simulink model "BaseEngineController_A02_bak2"  (Simulink 7.7)
settings: Solver=FixedStepDiscrete  StartTime=0.0  StopTime=10.0  RelTol=1e-3
PreLoadFcn:
  | try, eval([bdroot '_setup']); catch, eval('model_setup', ''); end
InitFcn:
  | try, eval([bdroot '_setup']); catch, eval('model_setup', ''); end
undefined symbols (not defined in the file or in its folder): ECU555, High, motohawk_build_version, motohawk_trunc_name, motohawk_vardec_config_group, motohawk_vardec_path, True
libraries: MotoHawk_lib x1081, AutoDoc_lib x609, motohawk_control_primitives_lib x132, motohawk_engine_lib x26, motohawk_annotations_lib x9, simulink x8, motohawk_control_primitives_motoshare_lib x1, simulink_extras x1
(part 6 of 22)

== system BaseEngineController_A02_bak2/Foreground/Control/VirtualSensors/AirCalcs ==
  RawIndexes : Inport
  Raw : Inport  Port=2
  SSEngOpr : Inport  Port=3
  RawProcessed : Inport  Port=4
  Baro Stall State Delay : Stateflow chart  TreatAsAtomicUnit=on
  Bus Creator1 : BusCreator  Inputs=2
  Bus Selector1 : BusSelector  OutputSignals=LinearSensors.MAP_Angular,RPM,NonLinearSensors.IAT,Digital.MAF
  Bus Selector2 : BusSelector  OutputSignals=ECT09Idx
  Bus Selector3 : BusSelector  OutputSignals=IAT17Idx,RPM17Idx
  Bus Selector4 : BusSelector  OutputSignals=NonLinearSensors.ECT
  Bus Selector5 : BusSelector  OutputSignals=LinearSensors.MAP_Time_Raw
  Bus Selector6 : BusSelector  OutputSignals=TimeSinceRun,EngineState
  Capture Signal At Startup : Subsystem  AncestorBlock=motohawk_engine_lib/Capture Signal At Startup  TreatAsAtomicUnit=on  mask(Capture Signal At Startup){nam='Baro', count=5, units='kPa', grp=motohawk_vardec_path(gcb)}
      mask prompts: nam "Signal Name"; count "Number Of Samples To Average"; grp "MotoTune GroupString Name"
      mask init:
      | lbl = sprintf('CAPTURE IN STALL\nAverage of\nfirst %d samples', count);
      mask Display:
      | patch([0 0 1 1], [0 0.075 0.075 0], [230/255 0/255 0/255])
      | patch([0 0 1 1], [0.025 0.05 0.05 0.025], [150/255 0/255 0/255])
      | patch([0 0 1 1], [0 0.025 0.025 0], [0/255 0/255 0/255])
      | 
      | disp(lbl);
  Documentation_Calibrations_Probes_Block : AutoDoc_lib/Documentation_Calibrations_Probes_Block
  Documentation_Left_Border : AutoDoc_lib/Documentation_Left_Border  layout=Portrait
  Documentation_Right_Border : AutoDoc_lib/Documentation_Right_Border  layout=Portrait
  Documentation_Title_Block : AutoDoc_lib/Documentation_Title_Block
  Saturation1 : Saturate  UpperLimit=inf  LowerLimit=1
  Speed Density - MAP Referenced - Adaptive : Subsystem  Description=|  AncestorBlock=motohawk_engine_lib/Speed Density - MAP Referenced - Adaptive
      | /* Name: PortPressRatio20IdxArr Unit:unitless ClassID:ORDARR StorageID:FLASH Access:RW4+RW3+NA2+NA1              
      |    TypeID:UINT2 Gain:0.0001 Min:0.0000 Max:6.5535 Format:5.4 UpdateID:REMOTE OrdLen:20 Group:"Engine Control | " 
      |    "Run | Airflow" Help:"Port pressure ratio index array. Used for speed-density flow computations." VardecSig:6E
      |    */                                                                                                            
      |                                                                                                                  
      | /* Name: RPM20IdxArr Unit:RPM20IdxArr ClassID:ORDARR StorageID:FLASH Access:RW4+RW3+NA2+NA1                      
      |    TypeID:UINT2 Gain:0.1 Min:0.0 Max:6553.0 Format:5.1 UpdateID:REMOTE OrdLen:20 Group:"Global "                 
      |    "Index Arrays | RPM" Help:"20 breakpoint RPM index array." VardecSig:5E */                                    
      |                                                                                                                  
      | /* Name: VolEffMap Unit:Unitless ClassID:MAP StorageID:FLASH Access:RW4+RW3+NA2+NA1 TypeID:UINT2                 
      |    Gain:0.0001 Min:0.00 Max:6.55 Format:6.2 UpdateID:REMOTE XOrdArr:PortPressRatio20IdxArr                       
      |    YOrdArr:RPM20IdxArr Group:"Engine Control | Run | Airflow" Help:"Engine volumetric efficency "                
      |    "map." VardecSig:61 */                                                                                        
      |                                                                                                                  
      | /* Name: IAT10IdxArr Unit:C ClassID:ORDARR StorageID:FLASH Access:RW4+RW3+NA2+NA1                                
      |    TypeID:SINT2 Gain:0.01 Min:-327.6800 Max:327.6700 Format:5.4 UpdateID:REMOTE OrdLen:10                        
      |    Group:"Engine Control | Run | Airflow" Help:"10 breakpoint intake air temperture index "                      
      |    "array." VardecSig:5C */                                                                                      
      |                                                                                                                  
      | /* Name: VETempCorrTbl ClassID:TBL StorageID:FLASH Access:RW4+RW3+NA2+NA1 TypeID:UINT2 Gain:0.0001 Min:0.00      
      |    Max:6.55 Format:4.2 UpdateID:REMOTE OrdArr:IAT10IdxArr Group:"Engine Control | Run | Airflow"                 
      |    Help:"Volumetric efficiency temperature correction table." VardecSig:6C */                                    
      |                                                                                                                  
      | /* Name: BaroPress10IdxArr Unit:C ClassID:ORDARR StorageID:FLASH Access:RW4+RW3+NA2+NA1                          
      |    TypeID:UINT2 Gain:0.01 Min:0.0000 Max:655.3500 Format:5.4 UpdateID:REMOTE OrdLen:10                           
      |    Group:"Engine Control | Run | Airflow" Help:"10 breakpoint barometric pressure index "                        
      |    "array." VardecSig:5C */                                                                                      
      |                                                                                                                  
      | /* Name: VEBaroCorrTbl ClassID:TBL StorageID:FLASH Access:RW4+RW3+NA2+NA1 TypeID:UINT2 Gain:0.0001 Min:0.00      
      |    Max:6.55 Format:4.2 UpdateID:REMOTE OrdArr:BaroPress10IdxArr Group:"Engine Control | Run | Airflow"           
      |    Help:"Volumetric efficiency barometric pressure correction table." VardecSig:6C */                            
      |                                                                                                                  
      | /* Name: CylSweptVol Unit:cm^3 ClassID:VAR StorageID:FLASH Access:RW4+RW3+NA2+NA1 TypeID:UINT2 Gain:0.1          
      |    Min:0.0 Max:6553.0 Format:5.1 UpdateID:REMOTE Group:"Engine Control | Run | Airflow" Help:"Cylinder swept "   
      |    "volume." VardecSig:6C */                                                                                     
      |                                                                                                                  
      | /* Name: MAFPort Unit:g/s ClassID:VAR StorageID:RAM Access:RO4+RO3+NA2+NA1 TypeID:UINT2 Gain:0.01                
      |    Min:0.00 Max:655.00 Format:5.2 UpdateID:BACKGND Group:"Engine Control | Run | Airflow" Help:"Total "          
      |    "ingested cylinder charge mass flow rate. The sum of all port flows. This values includes any EGR and "       
      |    "Purge mass flows." VardecSig:6A */                                                                           
      |                                                                                                                  
      | /* Name: CombEventsPerRev ClassID:VAR StorageID:FLASH Access:RW4+RW3+NA2+NA1 TypeID:UINT1 Gain:0.5 Min:0.5       
      |    Max:127.0 Format:4.1 UpdateID:REMOTE Group:"Engine Control | Run | Airflow" Help:"Number of conbustion "      
      |    "events per engine revolution. For example, for a four-stroke, eight cylinder engine the value would be 4."   
      |    VardecSig:6C */
      mask Description: Calculates air mass flow rate per cylinder. The estimated mass flow rate is that of the entire mixture: air, EGR and purge.
      mask Help: Given calibrations for the volumetric efficiency map, cylinder swept volume [cm3] and the VE temperature and pressure correction tables, the block calculates the air mass flow rate [g/s] per cylinder, given the barometric and manifold pressures [kPa], the manifold air temperature [C] and RPM.
      mask Display:
      | patch([0 0 1 1], 0.5*[0 0.075 0.075 0], [230/255 0/255 0/255])
      | patch([0 0 1 1], 0.5*[0.025 0.05 0.05 0.025], [150/255 0/255 0/255])
      | patch([0 0 1 1], 0.5*[0 0.025 0.025 0], [0/255 0/255 0/255])
      | 
      | disp('MAP-REFERENCED\nSPEED-DENSITY\nCOMPUTATION\n(adaptive)');
  motohawk_calibration : MotoHawk_lib/Calibration & Probing Blocks/motohawk_calibration  nam='BaroRunStallDelay'  val=4  storage=Calibration  show_more=on  name_source=Use Parameter  data_type=Inherit from 'Default Value'  read_level=1  write_level=1  ovr_cals=on  view_as=Number  enum_struct={'Disabled', 'Enabled'}  vector_style=Wide Row  use_help_units=on  help='Delay time after transitioning from Run to Stall before resampling baro.'  units='s'  use_header_enum=off  row_header_enum={}  col_header_enum={}  use_min_max=off  min_val=-inf  max_val=inf  use_precision=off  precision=''  gain=1  offset=0  exp=1  use_mototune_group=on  mototune_group=motohawk_vardec_path(gcb)
  motohawk_data_read1 : MotoHawk_lib/Data Storage Blocks/motohawk_data_read  source=Lookup By Name  structure=Scalar  operation=Read entire data structure at once  sz_vec=4  sz_row=2  sz_col=3  typ=uint8  nam='EngineState'  checkNull=on  struct_inst_name='Struct1'  struct_nam='MyStruct'
  motohawk_delta_time : MotoHawk_lib/Extra Development Blocks/motohawk_delta_time  samp_time=-1  init_val=.005  mode=Seconds (double)
  motohawk_override_rel : MotoHawk_lib/Calibration & Probing Blocks/motohawk_override_rel  SystemSampleTime=-1  parent_name='BARO'  show_more=on  name_source=Use Parameter  parent_read_level=1  parent_override_level=1  use_help_units=on  parent_help='Provides an override to the input by adding this amount to either the locked or running value'  parent_units='kPa'  use_min_max=off  parent_min=-inf  parent_max=inf  use_precision=off  parent_precision=''  parent_gain=1  parent_offset=0  parent_exp=1  use_mototune_group=on  parent_group=motohawk_vardec_path(gcb)
  motohawk_probe : MotoHawk_lib/Calibration & Probing Blocks/motohawk_probe  nam='BARO'  show_more=on  name_source=Use Parameter  read_level=1  view_as=Number  enum_struct={'Disabled', 'Enabled'}  vector_style=Wide Row  use_help_units=on  help=''  units='kPa'  use_header_enum=off  row_header_enum={}  col_header_enum={}  use_precision=off  precision=''  gain=1  offset=0  exp=1  use_mototune_group=on  mototune_group=motohawk_vardec_path(gcb)
  motohawk_probe1 : MotoHawk_lib/Calibration & Probing Blocks/motohawk_probe  nam='BaroDelayTime'  show_more=on  name_source=Use Parameter  read_level=1  view_as=Number  enum_struct={'Disabled', 'Enabled'}  vector_style=Wide Row  use_help_units=on  help=''  units='s'  use_header_enum=off  row_header_enum={}  col_header_enum={}  use_precision=off  precision=''  gain=1  offset=0  exp=1  use_mototune_group=on  mototune_group=motohawk_vardec_path(gcb)
  AirCalcs : Outport
  wires:
  Bus Selector5/1 -> Capture Signal At Startup/1(Signal_In)  "<MAP_Time_Raw>"
  Bus Selector1/3 -> Speed Density - MAP Referenced - Adaptive/4(IAT [C])  "<IAT>"
  Raw/1 -> Bus Selector5/1, Bus Selector1/1, Bus Selector4/1
  RawIndexes/1 -> Bus Selector3/1, Bus Selector2/1
  Bus Selector3/1 -> Speed Density - MAP Referenced - Adaptive/6(IAT17Idx)  "<IAT17Idx>"
  Bus Selector3/2 -> Speed Density - MAP Referenced - Adaptive/7(RPMIdx)  "<RPM17Idx>"
  Bus Selector1/1 -> Speed Density - MAP Referenced - Adaptive/2(MAP [kPa])  "<MAP_Angular>"
  Bus Selector1/2 -> Speed Density - MAP Referenced - Adaptive/3(RPM)  "<RPM>"
  Bus Creator1/1 -> AirCalcs/1  "AirCalcs"
  Speed Density - MAP Referenced - Adaptive/1(MAF [g/s]) -> Bus Creator1/2  "MAFPort"
  motohawk_override_rel/1 -> motohawk_probe/1, Bus Creator1/1, Saturation1/1  "Baro"
  Capture Signal At Startup/1(Signal_KeyUp) -> motohawk_override_rel/1  "Baro"
  Saturation1/1 -> Speed Density - MAP Referenced - Adaptive/1(BARO [kPa])
  Bus Selector1/4 -> Speed Density - MAP Referenced - Adaptive/5(MAF_Sensor [g/s])  "<MAF>"
  Baro Stall State Delay/2(Enable) -> Capture Signal At Startup/Enable
  motohawk_data_read1/1 -> Baro Stall State Delay/1(EngineState)
  motohawk_delta_time/1 -> Baro Stall State Delay/3(dT)
  motohawk_calibration/1 -> Baro Stall State Delay/2(TimeThresh)
  Baro Stall State Delay/1(Timer) -> motohawk_probe1/1
  SSEngOpr/1 -> Speed Density - MAP Referenced - Adaptive/8(SSEngOpr [bool])
  Bus Selector2/1 -> Speed Density - MAP Referenced - Adaptive/9(ECT09Idx)  "<ECT09Idx>"
  Bus Selector4/1 -> Speed Density - MAP Referenced - Adaptive/10(ECT [C])  "<ECT>"
  RawProcessed/1 -> Bus Selector6/1
  Bus Selector6/2 -> Speed Density - MAP Referenced - Adaptive/12(EngState)  "<EngineState>"
  Bus Selector6/1 -> Speed Density - MAP Referenced - Adaptive/11(TimeAfterRun [S])  "<TimeSinceRun>"

== system BaseEngineController_A02_bak2/Foreground/Control/VirtualSensors/LoadBasedCalcs ==
  RAW : Inport
  Bus Selector : BusSelector  OutputSignals=LinearSensors.MAP_Angular,RPM
  Engine Steady-State Flag : Subsystem  Description=|  AncestorBlock=motohawk_engine_lib/Engine Steady-State Flag  mask(Steady-State Engine Operation){samp_time=-1, group=motohawk_vardec_path(gcb)}
      | /* Name: SSTorqueFilterConst Unit:sec ClassID:VAR StorageID:FLASH Access:RW4+RW3+NA2+NA1 TypeID:UINT2            
      |    Gain:0.001 Min:0.00 Max:65.54 Format:4.2 UpdateID:REMOTE Group:"Engine Control | States" Help:"First-order "  
      |    "filter time constant used to filter torque. This value must be less than SSTorqueTol from unfiltered torque "
      |    "before the steady-state flag can be enabled." VardecSig:6C */                                                
      |                                                                                                                  
      | /* Name: SSRPMFilterConst Unit:sec ClassID:VAR StorageID:FLASH Access:RW4+RW3+NA2+NA1 TypeID:UINT2               
      |    Gain:0.001 Min:0.00 Max:65.54 Format:4.2 UpdateID:REMOTE Group:"Engine Control | States" Help:"First-order "  
      |    "filter time constant used to filter torque. This value must be less than SSRPMTol from unfiltered torque "   
      |    "before the steady-state flag can be enabled." VardecSig:6C */                                                
      |                                                                                                                  
      | /* Name: SSTorqueTol Unit:N.m ClassID:VAR StorageID:FLASH Access:RW4+RW3+NA2+NA1 TypeID:UINT2 Gain:0.001         
      |    Min:0.00 Max:65.54 Format:4.2 UpdateID:REMOTE Group:"Engine Control | States" Help:"Tolerance within which "  
      |    "the difference between filtered and unfiltered torque must lie before the steady-state flag can be "         
      |    "enabled." VardecSig:6C */                                                                                    
      |                                                                                                                  
      | /* Name: SSRPMTol Unit:RPM ClassID:VAR StorageID:FLASH Access:RW4+RW3+NA2+NA1 TypeID:UINT2 Gain:0.01             
      |    Min:0.00 Max:655.35 Format:4.2 UpdateID:REMOTE Group:"Engine Control | States" Help:"Tolerance within which " 
      |    "the difference between filtered and unfiltered RPM must lie before the steady-state flag can be enabled."    
      |    VardecSig:6C */
      mask prompts: samp_time "Sample Time [s]:"; group "MotoTune Group String"
      mask help:
      | This block determines whether or not the engine has achieved steady-state operation. It accomplishes this by applying a first-order low-pass filter to the indicated torque and RPM inputs. The filetrcoeficeints are individually calibratable for each. Once both the filtered torque and RPM values are within the calibratable toleraance of the input values, engine operation is assumed to be steady-state.
      |
      | If 'Sample Time' is -1, then extra code and RAM will be generated to dynamically calculate dt.
      mask Display:
      | patch([0 0 1 1], [0 0.075 0.075 0], [230/255 0/255 0/255])
      | patch([0 0 1 1], [0.025 0.05 0.05 0.025], [150/255 0/255 0/255])
      | patch([0 0 1 1], [0 0.025 0.025 0], [0/255 0/255 0/255])
      | 
      | disp('ENGINE\nSTEADY-STATE\nDETERMINATION')
  motohawk_probe : MotoHawk_lib/Calibration & Probing Blocks/motohawk_probe  nam='SSEng'  show_more=off  name_source=Use Parameter  read_level=1  view_as=Number  enum_struct={'Disabled', 'Enabled'}  vector_style=Wide Row  use_help_units=off  help=''  units=''  use_header_enum=off  row_header_enum={}  col_header_enum={}  use_precision=off  precision=''  gain=1  offset=0  exp=1  use_mototune_group=off  mototune_group=motohawk_vardec_path(gcb)
  SSEng : Outport
  wires:
  Engine Steady-State Flag/1(SSEngOpr [bool]) -> SSEng/1, motohawk_probe/1  "SSEng"
  RAW/1 -> Bus Selector/1
  Bus Selector/1 -> Engine Steady-State Flag/1(Load)  "<MAP_Angular>"
  Bus Selector/2 -> Engine Steady-State Flag/2(RPM)  "<RPM>"

== system BaseEngineController_A02_bak2/Foreground/Control/VirtualSensors/Raw Indexes ==
  Raw : Inport
  TPSPcnt : Inport  Port=2
  ACPres Index : Subsystem
  Bus Creator1 : BusCreator  Inputs=15
  Bus Selector1 : BusSelector  OutputSignals=LinearSensors.ACPres
  Bus Selector2 : BusSelector  OutputSignals=LinearSensors.SysVolt
  Bus Selector3 : BusSelector  OutputSignals=LinearSensors.MAP_Angular
  Bus Selector4 : BusSelector  OutputSignals=Digital.VSPD
  Bus Selector5 : BusSelector  OutputSignals=NonLinearSensors.CAT
  Bus Selector6 : BusSelector  OutputSignals=RPM
  Bus Selector7 : BusSelector  OutputSignals=NonLinearSensors.IAT
  Bus Selector8 : BusSelector  OutputSignals=NonLinearSensors.ECT
  CAT Index : Subsystem
  Documentation_Calibrations_Probes_Block : AutoDoc_lib/Documentation_Calibrations_Probes_Block
  Documentation_Left_Border : AutoDoc_lib/Documentation_Left_Border  layout=Portrait
  Documentation_Right_Border : AutoDoc_lib/Documentation_Right_Border  layout=Portrait
  Documentation_Title_Block : AutoDoc_lib/Documentation_Title_Block
  ECT Index : Subsystem
  IAT Indexes : Subsystem
  MAP Indexes : Subsystem
  RPM Indexes : Subsystem
  SysVolt Index : Subsystem
  TPS Indexes : Subsystem
  VSPD Index : Subsystem
  RawIndexes : Outport
  wires:
  RPM Indexes/1(RPM05Idx) -> Bus Creator1/1  "RPM05Idx"
  RPM Indexes/2(RPM09Idx) -> Bus Creator1/2  "RPM09Idx"
  TPS Indexes/1(TPS09Idx) -> Bus Creator1/4  "TPS09Idx"
  Bus Selector7/1 -> IAT Indexes/1(IAT)  "<IAT>"
  ECT Index/1(ECT05Idx) -> Bus Creator1/5  "ECT05Idx"
  Bus Selector8/1 -> ECT Index/1(ECT)  "<ECT>"
  Bus Creator1/1 -> RawIndexes/1  "RawIndexes"
  Bus Selector6/1 -> RPM Indexes/1(RPM)  "<RPM>"
  Raw/1 -> Bus Selector6/1, Bus Selector8/1, Bus Selector7/1, Bus Selector2/1, Bus Selector1/1, Bus Selector3/1, Bus Selector4/1, Bus Selector5/1
  Bus Selector2/1 -> SysVolt Index/1(SysVolt)  "<SysVolt>"
  SysVolt Index/1(SysVolt09Idx) -> Bus Creator1/9  "SysVolt09Idx"
  RPM Indexes/3(RPM17Idx) -> Bus Creator1/3  "RPM17Idx"
  TPSPcnt/1 -> TPS Indexes/1(TPSPcnt)
  Bus Selector1/1 -> ACPres Index/1(ACPres)  "<ACPres>"
  ACPres Index/1(ACPres05Idx) -> Bus Creator1/10  "ACPres05Idx"
  Bus Selector3/1 -> MAP Indexes/1(MAP)  "<MAP_Angular>"
  MAP Indexes/1(MAP05Idx) -> Bus Creator1/11  "MAP05Idx"
  MAP Indexes/2(MAP09Idx) -> Bus Creator1/12  "MAP09Idx"
  MAP Indexes/3(MAP17Idx) -> Bus Creator1/13  "MAP17Idx"
  IAT Indexes/2(IAT17Idx) -> Bus Creator1/8  "IAT17Idx"
  IAT Indexes/1(IAT05Idx) -> Bus Creator1/7  "IAT05Idx"
  ECT Index/2(ECT09Idx) -> Bus Creator1/6  "ECT09Idx"
  Bus Selector4/1 -> VSPD Index/1(VSPD)  "<VSPD>"
  VSPD Index/1(VSPD09Idx) -> Bus Creator1/14  "VSPD09Idx"
  Bus Selector5/1 -> CAT Index/1(CAT)  "<CAT>"
  CAT Index/1(CAT05Idx) -> Bus Creator1/15  "CAT05Idx"

== system BaseEngineController_A02_bak2/Foreground/Control/VirtualSensors/RawProcessed ==
  Raw : Inport
  Indexes : Inport  Port=2
  APP  Sensor Arbitration1 : Subsystem
      mask Display:
      | patch([0 0 1 1], [0 0.075 0.075 0], [230/255 0/255 0/255])
      | patch([0 0 1 1], [0.025 0.05 0.05 0.025], [150/255 0/255 0/255])
      | patch([0 0 1 1], [0 0.025 0.025 0], [0/255 0/255 0/255])
      | 
      | disp('APPSensor\nArbitration');
  Base TPS Request : Subsystem  TreatAsAtomicUnit=on
  Bus Creator : BusCreator  Inputs=2
  Bus Creator2 : BusCreator  Inputs=15
  Bus Selector1 : BusSelector  OutputSignals=PotSensors.TPS2
  Bus Selector10 : BusSelector  OutputSignals=PotSensors.TPS1
  Bus Selector11 : BusSelector  OutputSignals=NonLinearSensors.O2_PreCat
  Bus Selector12 : BusSelector  OutputSignals=RPM
  Bus Selector2 : BusSelector  OutputSignals=RPMInst
  Bus Selector3 : BusSelector  OutputSignals=LinearSensors.FuelPres,LinearSensors.Map_Time
  Bus Selector4 : BusSelector  OutputSignals=PotSensors.APP1,PotSensors.APP2
  Bus Selector5 : BusSelector  OutputSignals=NonLinearSensors.ECT_Raw
  Bus Selector6 : BusSelector  OutputSignals=TPS09Idx
  Bus Selector7 : BusSelector  OutputSignals=NonLinearSensors.IAT_Raw
  Capture ECT At Startup : Subsystem  TreatAsAtomicUnit=on  mask(Capture Signal At Startup){nam='ECT_KeyUp', count=5, units='C', grp=motohawk_vardec_path(gcb)}
      mask prompts: nam "Signal Name"; count "Number Of Samples To Average"; grp "MotoTune GroupString Name"
      mask init:
      | lbl = sprintf('CAPTURE IN STALL\nAverage of\nfirst %d samples', count);
      mask Display:
      | patch([0 0 1 1], [0 0.075 0.075 0], [230/255 0/255 0/255])
      | patch([0 0 1 1], [0.025 0.05 0.05 0.025], [150/255 0/255 0/255])
      | patch([0 0 1 1], [0 0.025 0.025 0], [0/255 0/255 0/255])
      | 
      | disp(lbl);
  Capture IAT At Startup : Subsystem  TreatAsAtomicUnit=on  mask(Capture Signal At Startup){nam='IAT_KeyUp', count=5, units='C', grp='Sensors | IAT'}
      mask prompts: nam "Signal Name"; count "Number Of Samples To Average"; grp "MotoTune GroupString Name"
      mask init:
      | lbl = sprintf('CAPTURE IN STALL\nAverage of\nfirst %d samples', count);
      mask Display:
      | patch([0 0 1 1], [0 0.075 0.075 0], [230/255 0/255 0/255])
      | patch([0 0 1 1], [0.025 0.05 0.05 0.025], [150/255 0/255 0/255])
      | patch([0 0 1 1], [0 0.025 0.025 0], [0/255 0/255 0/255])
      | 
      | disp(lbl);
  CutThrottle APP Limiting : Subsystem
      mask Display:
      | patch([0 0 1 1], [0 0.075 0.075 0], [230/255 0/255 0/255])
      | patch([0 0 1 1], [0.025 0.05 0.05 0.025], [150/255 0/255 0/255])
      | patch([0 0 1 1], [0 0.025 0.025 0], [0/255 0/255 0/255])
      | 
      | disp('');
  Documentation_Calibrations_Probes_Block : AutoDoc_lib/Documentation_Calibrations_Probes_Block
  Documentation_Left_Border : AutoDoc_lib/Documentation_Left_Border  layout=Portrait
  Documentation_Right_Border : AutoDoc_lib/Documentation_Right_Border  layout=Portrait
  Documentation_Title_Block : AutoDoc_lib/Documentation_Title_Block
  Engine Accel : Subsystem
  EngineState : Subsystem
      mask Display: disp('ENGINE STATE\nDETERMINATION\n\n1 - Stall\n2 - Crank\n3 - Run');
  Fuel Delta Pressure Calculation : Subsystem
      mask Display:
      | patch([0 0 1 1], [0 0.075 0.075 0], [230/255 0/255 0/255])
      | patch([0 0 1 1], [0.025 0.05 0.05 0.025], [150/255 0/255 0/255])
      | patch([0 0 1 1], [0 0.025 0.025 0], [0/255 0/255 0/255])
      | 
      | disp('Fuel Pressure \n Across Injectors');
  O2 De-lag : Subsystem
  Stall : motohawk_control_primitives_lib/CompareTo  SystemSampleTime=-1  op===  val=1
  TPS  Sensor Arbitration : Subsystem
      mask Display:
      | patch([0 0 1 1], [0 0.075 0.075 0], [230/255 0/255 0/255])
      | patch([0 0 1 1], [0.025 0.05 0.05 0.025], [150/255 0/255 0/255])
      | patch([0 0 1 1], [0 0.025 0.025 0], [0/255 0/255 0/255])
      | 
      | disp('TPS Sensor\nArbitration');
  Time Since Key On : Subsystem
  Time Since Run : Subsystem
  Time Since Stall : Subsystem
  motohawk_data_read : MotoHawk_lib/Data Storage Blocks/motohawk_data_read  source=Lookup By Name  structure=Scalar  operation=Read entire data structure at once  sz_vec=4  sz_row=2  sz_col=3  typ=uint8  nam='EngineState'  checkNull=on  struct_inst_name='Struct1'  struct_nam='MyStruct'
  motohawk_probe : MotoHawk_lib/Calibration & Probing Blocks/motohawk_probe  nam='APPPcnt'  show_more=on  name_source=Use Parameter  read_level=1  view_as=Number  enum_struct={'Disabled', 'Enabled'}  vector_style=Wide Row  use_help_units=on  help='APP used in controller (after power curve and CutThrottle limiting'  units='%'  use_header_enum=off  row_header_enum={}  col_header_enum={}  use_precision=on  precision=''  gain=1  offset=0  exp=1  use_mototune_group=on  mototune_group=motohawk_vardec_path(gcb)
  RawProcessed : Outport
  wires:
  Base TPS Request/3(APP Idx) -> Bus Creator2/6  "APPIdx"
  Bus Selector7/1 -> Capture IAT At Startup/1(Signal_In)  "<IAT_Raw>"
  Capture IAT At Startup/1(Signal_KeyUp) -> Bus Creator2/15  "IAT_KeyUp"
  Bus Selector2/1 -> EngineState/1(RPM)  "<RPMInst>"
  Bus Selector12/1 -> Engine Accel/1(RPM)  "<RPM>"
  EngineState/1(State) -> Bus Creator2/1  "EngineState"
  Base TPS Request/1(ETCReqFilt) -> Bus Creator2/4  "ETCRequest"
  Engine Accel/1(RPM Accel) -> Bus Creator2/9  "RPM_Accel"
  Bus Creator2/1 -> RawProcessed/1  "RawProcessed"
  Raw/1 -> Bus Selector11/1, Bus Selector12/1, Bus Selector10/1, Bus Selector7/1, Bus Selector5/1, Bus Selector1/1, Bus Selector3/1, Bus Selector4/1, Bus Selector2/1
  Bus Selector10/1 -> TPS  Sensor Arbitration/1(TPS1)  "<TPS1>"
  Bus Selector1/1 -> TPS  Sensor Arbitration/2(TPS2)  "<TPS2>"
  TPS  Sensor Arbitration/1(TPS) -> Bus Creator2/10  "TPS"
  Bus Selector5/1 -> Capture ECT At Startup/1(Signal_In)  "<ECT_Raw>"
  motohawk_data_read/1 -> Stall/1
  Stall/1 -> Capture IAT At Startup/Enable, Capture ECT At Startup/Enable
  Capture ECT At Startup/1(Signal_KeyUp) -> Bus Creator2/11  "ECT_KeyUp"
  Base TPS Request/2(ETCReqRaw) -> Bus Creator2/5  "ETCReqRaw"
  Time Since Stall/1(Time) -> Bus Creator2/12  "TimeSinceStall"
  Time Since Run/1(Time) -> Bus Creator2/13  "TimeSinceRun"
  Bus Selector6/1 -> Base TPS Request/2(TPS_Idx)  "<TPS09Idx>"
  Indexes/1 -> Bus Selector6/1
  Time Since Key On/1(Time) -> Bus Creator2/14  "TimeSinceKeyOn"
  Bus Selector11/1 -> O2 De-lag/2(PostCat), O2 De-lag/1(PreCat)  "<O2_PreCat>"
  Bus Creator/1 -> Bus Creator2/8  "O2Delagged"
  O2 De-lag/1(PreCatDelagged) -> Bus Creator/1  "PreO2Delag"
  O2 De-lag/2(PostCatDelagged) -> Bus Creator/2  "PostO2Delag"
  Bus Selector3/1 -> Fuel Delta Pressure Calculation/1(FuelPressure)  "<FuelPres>"
  Bus Selector3/2 -> Fuel Delta Pressure Calculation/2("MAP ")  "<Map_Time>"
  Fuel Delta Pressure Calculation/1("FuelDeltaPressure ") -> Bus Creator2/7  "FuelDeltaPressure"
  CutThrottle APP Limiting/1(APP_out [%]) -> Bus Creator2/2, motohawk_probe/1, Base TPS Request/1(APP Pcnt)  "APPPcnt"
  Bus Selector4/1 -> APP  Sensor Arbitration1/1(APP1)  "<APP1>"
  Bus Selector4/2 -> APP  Sensor Arbitration1/2(APP2)  "<APP2>"
  APP  Sensor Arbitration1/1(APP) -> CutThrottle APP Limiting/1(APP_in [%]), Bus Creator2/3  "APP1"

== system BaseEngineController_A02_bak2/Foreground/Control/VirtualSensors/Virtual Indexes ==
  AirCalcs : Inport
  RawProcessed : Inport  Port=2
  Bus Creator2 : BusCreator  Inputs=6
  Bus Selector3 : BusSelector  OutputSignals=MAFPort
  Bus Selector4 : BusSelector  OutputSignals=ECT_KeyUp
  Bus Selector5 : BusSelector  OutputSignals=RPM_Accel
  Bus Selector6 : BusSelector  OutputSignals=APP1
  Bus Selector7 : BusSelector  OutputSignals=TimeSinceRun
  Bus Selector8 : BusSelector  OutputSignals=IAT_KeyUp
  Documentation_Calibrations_Probes_Block : AutoDoc_lib/Documentation_Calibrations_Probes_Block
  Documentation_Left_Border : AutoDoc_lib/Documentation_Left_Border  layout=Portrait
  Documentation_Right_Border : AutoDoc_lib/Documentation_Right_Border  layout=Portrait
  Documentation_Title_Block : AutoDoc_lib/Documentation_Title_Block
  ECT_KeyUp : Subsystem
  IAT_KeyUp : Subsystem
  MAFPortIndex : Subsystem
  motohawk_prelookup : MotoHawk_lib/Lookup Tables/motohawk_prelookup  nam='RPMAccel17'  breakpoint_data=ones(1,17)  show_more=on  data_type=Inherit from 'Table Data'  storage=Constant  read_level=1  write_level=1  ovr_cals=on  use_help=on  help=''  units=''  use_min_max=off  min_val=-inf  max_val=inf  use_precision=on  precision=''  gain=1  offset=0  exp=1  use_links=off  input_name=''  use_mototune_group=on  mototune_group=motohawk_vardec_path(gcb)
  motohawk_prelookup1 : MotoHawk_lib/Lookup Tables/motohawk_prelookup  nam='App05'  breakpoint_data=[0 50 70 90 100]  show_more=on  data_type=Inherit from 'Table Data'  storage=Constant  read_level=1  write_level=1  ovr_cals=on  use_help=on  help=''  units=''  use_min_max=off  min_val=-inf  max_val=inf  use_precision=on  precision=''  gain=1  offset=0  exp=1  use_links=off  input_name=''  use_mototune_group=on  mototune_group=motohawk_vardec_path(gcb)
  motohawk_prelookup2 : MotoHawk_lib/Lookup Tables/motohawk_prelookup  nam='TimeSinceRun09'  breakpoint_data=[0 2 4 6 10 15 20 30 45]  show_more=on  data_type=Inherit from 'Table Data'  storage=Constant  read_level=1  write_level=1  ovr_cals=on  use_help=on  help=''  units=''  use_min_max=off  min_val=-inf  max_val=inf  use_precision=on  precision=''  gain=1  offset=0  exp=1  use_links=off  input_name=''  use_mototune_group=on  mototune_group=motohawk_vardec_path(gcb)
  VirtualIndexes : Outport
  wires:
  Bus Creator2/1 -> VirtualIndexes/1  "Virtual Indexes"
  AirCalcs/1 -> Bus Selector3/1
  Bus Selector3/1 -> MAFPortIndex/1(MAFPort)  "<MAFPort>"
  Bus Selector4/1 -> ECT_KeyUp/1(ECT_KeyUp)  "<ECT_KeyUp>"
  RawProcessed/1 -> Bus Selector4/1, Bus Selector5/1, Bus Selector6/1, Bus Selector7/1, Bus Selector8/1
  Bus Selector5/1 -> motohawk_prelookup/1  "<RPM_Accel>"
  Bus Selector6/1 -> motohawk_prelookup1/1  "<APP1>"
  Bus Selector7/1 -> motohawk_prelookup2/1  "<TimeSinceRun>"
  Bus Selector8/1 -> IAT_KeyUp/1(IAT_KeyUp)  "<IAT_KeyUp>"
  MAFPortIndex/1(MAFPort05Idx) -> Bus Creator2/1  "MAFPort05Idx"
  ECT_KeyUp/1(ECT_KeyUp09Idx) -> Bus Creator2/2  "ECTKeyUp09Idx"
  motohawk_prelookup/1 -> Bus Creator2/3  "RPMAccel17Idx"
  motohawk_prelookup1/1 -> Bus Creator2/4  "App05Idx"
  motohawk_prelookup2/1 -> Bus Creator2/5  "TimeSinceRun09"
  IAT_KeyUp/1(IAT_KeyUp09Idx) -> Bus Creator2/6  "IATKeyUp09Idx"

== system BaseEngineController_A02_bak2/Foreground/Inputs/Digital/Scramble  ==
  ADC : Inport
  0 = No Inversion 1 = Inversion : MotoHawk_lib/Calibration & Probing Blocks/motohawk_calibration  nam=[prefix 'Polarity']  val=boolean(polarity-1)  storage=Calibration  show_more=on  name_source=Use Parameter  data_type=Inherit from 'Default Value'  read_level=1  write_level=1  ovr_cals=on  view_as=Number  enum_struct={'Disabled', 'Enabled'}  vector_style=Wide Row  use_help_units=on  help="'0 = High True    1 = Low True'"  units='bool'  use_header_enum=off  row_header_enum={}  col_header_enum={}  use_min_max=off  min_val=-inf  max_val=inf  use_precision=off  precision=''  gain=1  offset=0  exp=1  use_mototune_group=on  mototune_group=[group ' | ' nam ' Config']
  Button Delay 1 : Subsystem
  Data Type Conversion : DataTypeConversion  SaturateOnIntegerOverflow=on  RndMeth=Zero
  Logical Operator : Logic  Operator=XOR
  Override in Engineering Units : MotoHawk_lib/Calibration & Probing Blocks/motohawk_override_abs  SystemSampleTime=-1  parent_name=nam  parent_val=0  show_more=on  name_source=Use Parameter  parent_read_level=1  parent_override_level=1  view_as=Number  enum_struct={'Disabled', 'Enabled'}  use_help_units=on  parent_help='Override actuator, in engineering units'  parent_units='Boolean'  use_min_max=off  parent_min=-inf  parent_max=inf  use_precision=off  parent_precision=''  parent_gain=1  parent_offset=0  parent_exp=1  use_mototune_group=on  parent_group=group
  Relational Operator : RelationalOperator  Operator=>
  SwitchPoint : MotoHawk_lib/Calibration & Probing Blocks/motohawk_calibration  nam=[prefix 'SwitchPt']  val=switchpoint  storage=Calibration  show_more=on  name_source=Use Parameter  data_type=Inherit from 'Default Value'  read_level=1  write_level=1  ovr_cals=on  view_as=Number  enum_struct={'Disabled', 'Enabled'}  vector_style=Wide Row  use_help_units=on  help='Defines switch point in ADC units boolean conversion'  units='ADC'  use_header_enum=off  row_header_enum={}  col_header_enum={}  use_min_max=off  min_val=-inf  max_val=inf  use_precision=off  precision=''  gain=1  offset=0  exp=1  use_mototune_group=on  mototune_group=[group ' | ' nam ' Config']
  motohawk_calibration7 : MotoHawk_lib/Calibration & Probing Blocks/motohawk_calibration  nam='ScrambleDebounceDelay'  val=0.08  storage=Calibration  show_more=on  name_source=Use Parameter  data_type=Inherit from 'Default Value'  read_level=1  write_level=1  ovr_cals=on  view_as=Number  enum_struct={'Disabled', 'Enabled'}  vector_style=Wide Row  use_help_units=on  help='Cruise control debounce time'  units='S'  use_header_enum=off  row_header_enum={}  col_header_enum={}  use_min_max=off  min_val=-inf  max_val=inf  use_precision=off  precision=''  gain=1  offset=0  exp=1  use_mototune_group=off  mototune_group=motohawk_vardec_path(gcb)
  motohawk_probe : MotoHawk_lib/Calibration & Probing Blocks/motohawk_probe  nam=nam  show_more=on  name_source=Use Parameter  read_level=1  view_as=Number  enum_struct={'Disabled', 'Enabled'}  vector_style=Wide Row  use_help_units=on  help='Display output in engineering units'  units='Boolean'  use_header_enum=off  row_header_enum={}  col_header_enum={}  use_precision=off  precision=''  gain=1  offset=0  exp=1  use_mototune_group=on  mototune_group=group
  motohawk_probe1 : MotoHawk_lib/Calibration & Probing Blocks/motohawk_probe  nam=[nam '_Raw']  show_more=on  name_source=Use Parameter  read_level=1  view_as=Number  enum_struct={'Disabled', 'Enabled'}  vector_style=Wide Row  use_help_units=on  help='Display output in engineering units'  units='ADC'  use_header_enum=off  row_header_enum={}  col_header_enum={}  use_precision=off  precision=''  gain=1  offset=0  exp=1  use_mototune_group=on  mototune_group=group
  DiscOut : Outport
  wires:
  ADC/1 -> Data Type Conversion/1
  0 = No Inversion 1 = Inversion/1 -> Logical Operator/2
  Relational Operator/1 -> Logical Operator/1
  SwitchPoint/1 -> Relational Operator/2
  Override in Engineering Units/1 -> DiscOut/1, motohawk_probe/1
  Data Type Conversion/1 -> Relational Operator/1, motohawk_probe1/1
  motohawk_calibration7/1 -> Button Delay 1/2(Time)
  Logical Operator/1 -> Button Delay 1/1(In1)
  Button Delay 1/1(Out1) -> Override in Engineering Units/1

== system BaseEngineController_A02_bak2/Foreground/Inputs/Digital/Two Step ==
  Boolean : Inport
  0 = No Inversion 1 = Inversion : MotoHawk_lib/Calibration & Probing Blocks/motohawk_calibration  nam=[prefix 'Polarity']  val=boolean(polarity-1)  storage=Calibration  show_more=on  name_source=Use Parameter  data_type=Inherit from 'Default Value'  read_level=1  write_level=1  ovr_cals=on  view_as=Number  enum_struct={'Disabled', 'Enabled'}  vector_style=Wide Row  use_help_units=on  help="'0 = High True    1 = Low True'"  units='bool'  use_header_enum=off  row_header_enum={}  col_header_enum={}  use_min_max=off  min_val=-inf  max_val=inf  use_precision=off  precision=''  gain=1  offset=0  exp=1  use_mototune_group=on  mototune_group=[group ' | ' nam ' Config']
  Button Delay 1 : Subsystem
  Logical Operator : Logic  Operator=XOR
  Override in Engineering Units : MotoHawk_lib/Calibration & Probing Blocks/motohawk_override_abs  SystemSampleTime=-1  parent_name=nam  parent_val=0  show_more=on  name_source=Use Parameter  parent_read_level=1  parent_override_level=1  view_as=Number  enum_struct={'Disabled', 'Enabled'}  use_help_units=on  parent_help='Override actuator, in engineering units'  parent_units='Boolean'  use_min_max=off  parent_min=-inf  parent_max=inf  use_precision=off  parent_precision=''  parent_gain=1  parent_offset=0  parent_exp=1  use_mototune_group=on  parent_group=group
  motohawk_calibration7 : MotoHawk_lib/Calibration & Probing Blocks/motohawk_calibration  nam='TwoStepDebounceDelay'  val=0.08  storage=Calibration  show_more=on  name_source=Use Parameter  data_type=Inherit from 'Default Value'  read_level=1  write_level=1  ovr_cals=on  view_as=Number  enum_struct={'Disabled', 'Enabled'}  vector_style=Wide Row  use_help_units=on  help='Two step debounce time'  units='S'  use_header_enum=off  row_header_enum={}  col_header_enum={}  use_min_max=off  min_val=-inf  max_val=inf  use_precision=off  precision=''  gain=1  offset=0  exp=1  use_mototune_group=off  mototune_group=motohawk_vardec_path(gcb)
  motohawk_probe : MotoHawk_lib/Calibration & Probing Blocks/motohawk_probe  nam=nam  show_more=on  name_source=Use Parameter  read_level=1  view_as=Number  enum_struct={'Disabled', 'Enabled'}  vector_style=Wide Row  use_help_units=on  help='Display output in engineering units'  units='Boolean'  use_header_enum=off  row_header_enum={}  col_header_enum={}  use_precision=off  precision=''  gain=1  offset=0  exp=1  use_mototune_group=on  mototune_group=group
  motohawk_probe1 : MotoHawk_lib/Calibration & Probing Blocks/motohawk_probe  nam=[nam '_Raw']  show_more=on  name_source=Use Parameter  read_level=1  view_as=Number  enum_struct={'Disabled', 'Enabled'}  vector_style=Wide Row  use_help_units=on  help='Display output in engineering units'  units='Boolean'  use_header_enum=off  row_header_enum={}  col_header_enum={}  use_precision=off  precision=''  gain=1  offset=0  exp=1  use_mototune_group=on  mototune_group=group
  DiscOut : Outport
  wires:
  Override in Engineering Units/1 -> motohawk_probe/1, DiscOut/1
  0 = No Inversion 1 = Inversion/1 -> Logical Operator/2
  Boolean/1 -> Logical Operator/1, motohawk_probe1/1
  motohawk_calibration7/1 -> Button Delay 1/2(Time)
  Logical Operator/1 -> Button Delay 1/1(In1)
  Button Delay 1/1(Out1) -> Override in Engineering Units/1

== system BaseEngineController_A02_bak2/Foreground/Inputs/EngineSpeed/Encoder Diagnostics ==
  Encoder Fault : Inport
  CamEdges : motohawk_control_primitives_lib/CompareTo  SystemSampleTime=-1  op===  val=4
  CamSync : motohawk_control_primitives_lib/CompareTo  SystemSampleTime=-1  op===  val=5
  CounterThresh : motohawk_control_primitives_lib/CompareTo  SystemSampleTime=-1  op=>  val=100
  CrankEdges : motohawk_control_primitives_lib/CompareTo  SystemSampleTime=-1  op===  val=3
  CrankSync : motohawk_control_primitives_lib/CompareTo  SystemSampleTime=-1  op===  val=1
  Documentation_Calibrations_Probes_Block : AutoDoc_lib/Documentation_Calibrations_Probes_Block
  Documentation_Left_Border : AutoDoc_lib/Documentation_Left_Border  layout=Portrait
  Documentation_Right_Border : AutoDoc_lib/Documentation_Right_Border  layout=Portrait
  Documentation_Title_Block : AutoDoc_lib/Documentation_Title_Block
  Logical Operator{,1..3} (4 alike) : Logic  AllPortsSameDT=off  OutDataTypeStr=boolean
  motohawk_encoder_fault_trigger : MotoHawk_lib/Encoder Blocks/motohawk_encoder_fault_trigger  Tag=Trigger  inherited=on
  motohawk_fault_def : MotoHawk_lib/Fault Management Blocks/motohawk_fault_def  nam='CrankSyncFault'  mode=Enabled  id=[]  x=25  y=50  input_suspected=on  indeterminate=off  increment_xy=on  downsample_count=1  ovr_cals=on  ovr_actions=on  show_actions=off  action1_name=''  action1_cond=(None)  action2_name=''  action2_cond=(None)  action3_name=''  action3_cond=(None)  action4_name=''  action4_cond=(None)
  motohawk_fault_def1 : MotoHawk_lib/Fault Management Blocks/motohawk_fault_def  nam='CrankEdgesFault'  mode=Enabled  id=[]  x=25  y=50  input_suspected=on  indeterminate=off  increment_xy=on  downsample_count=1  ovr_cals=on  ovr_actions=on  show_actions=off  action1_name=''  action1_cond=(None)  action2_name=''  action2_cond=(None)  action3_name=''  action3_cond=(None)  action4_name=''  action4_cond=(None)
  motohawk_fault_def2 : MotoHawk_lib/Fault Management Blocks/motohawk_fault_def  nam='CamEdgesFault'  mode=Enabled  id=[]  x=25  y=50  input_suspected=on  indeterminate=off  increment_xy=on  downsample_count=1  ovr_cals=on  ovr_actions=on  show_actions=off  action1_name=''  action1_cond=(None)  action2_name=''  action2_cond=(None)  action3_name=''  action3_cond=(None)  action4_name=''  action4_cond=(None)
  motohawk_fault_def3 : MotoHawk_lib/Fault Management Blocks/motohawk_fault_def  nam='CamSyncFault'  mode=Enabled  id=[]  x=25  y=50  input_suspected=on  indeterminate=off  increment_xy=on  downsample_count=1  ovr_cals=on  ovr_actions=on  show_actions=off  action1_name=''  action1_cond=(None)  action2_name=''  action2_cond=(None)  action3_name=''  action3_cond=(None)  action4_name=''  action4_cond=(None)
  motohawk_model_probe_eval_f : Subsystem  AncestorBlock=MotoHawk_lib/Extra Development Blocks/motohawk_model_probe_eval_f  TreatAsAtomicUnit=on  mask(MotoHawk Debug Probe){nam='EncoderFaultCount', group=motohawk_vardec_path(gcb)}
      mask prompts: nam "Probe Name:"; group "MotoTune Group:"
      mask help:
      | Drops in a simple counter, visible from MotoTune, to see if the current subsystem is executing.
      |
      | The default expression for the Probe Name uses the name of the current subsystem (without spaces).
      |
      | ---------------------------------------------------------------------
      | Copyright 2005 MotoTron, Inc.  All Rights Reserved.
      mask init:
      | lbl = ['Debug Counter\nName: ' nam];
      mask Help: eval('motohawk_block_help(get_param(gcb,''MaskType''))');
      mask Display:
      | patch([0 0 1 1], [0 1 1 0], [220/255 220/255 220/255])
      | patch([0 0 1 1], [0 0.075 0.075 0], [230/255 0/255 0/255])
      | patch([0 0 1 1], [0.025 0.05 0.05 0.025], [150/255 0/255 0/255])
      | patch([0 0 1 1], [0 0.025 0.025 0], [0/255 0/255 0/255])
      | 
      | disp(lbl);
  motohawk_probe3 : MotoHawk_lib/Calibration & Probing Blocks/motohawk_probe  nam='EncoderFault'  show_more=on  name_source=Use Parameter  read_level=1  view_as=Enumeration  enum_struct={'No Fault','Crank Sync Fault','Reverse Rotation Fault','No Crank Edges Fault','No CAM edges fault','Cam Sync Fault'}  vector_style=Wide Row  use_help_units=off  help=''  units=''  use_header_enum=off  row_header_enum={}  col_header_enum={}  use_precision=off  precision=''  gain=1  offset=0  exp=1  use_mototune_group=on  mototune_group=motohawk_vardec_path(gcb)
  wires:
  Logical Operator3/1 -> motohawk_fault_def3/1
  CamSync/1 -> Logical Operator3/2
  Logical Operator2/1 -> motohawk_fault_def2/1
  CamEdges/1 -> Logical Operator2/2
  Logical Operator1/1 -> motohawk_fault_def1/1
  CrankEdges/1 -> Logical Operator1/2
  Encoder Fault/1 -> CamSync/1, CamEdges/1, CrankEdges/1, motohawk_probe3/1, CrankSync/1
  motohawk_encoder_fault_trigger/1 -> motohawk_model_probe_eval_f/Trigger
  motohawk_model_probe_eval_f/1(Count) -> CounterThresh/1
  Logical Operator/1 -> motohawk_fault_def/1
  CrankSync/1 -> Logical Operator/2
  CounterThresh/1 -> Logical Operator3/1, Logical Operator2/1, Logical Operator1/1, Logical Operator/1
